simulink model "TosAeb"  (R2021a)
settings: Solver=FixedStepDiscrete  StartTime=0.0  StopTime=inf  FixedStep=0.01  RelTol=1e-3
undefined symbols (not defined in the file or in its folder): ACCModSts, AccSts1, AEB_ObjectMovingOrientation, AEB_ObjectMovingState, CN_BTCActive, CN_CurrentGearR, CN_CurrentGearR_DHT, CN_PTCActive, CN_VDCActive, CN_VSC_EspOff, CN_VSC_FCW_AEB_Error, CN_VSC_FCW_AEB_NoDispay, CN_VSC_FCW_AEB_PerfmDgdtn, CN_VSC_FCW_AEB_TempUnAvlbl, CN_VSC_VehStandstill, CNaccelAvoidMax, CNaccelAvoidMin, CNbrakePedalPress, CNcomfortStrLeftAccelMax, CNcomfortStrLeftAccelMin, CNcomfortStrRightAccelMax, CNcomfortStrRightAccelMin, CNDrvDoorOpen, CNDrvModeEco, CNDrvModeNorm, CNDrvModeOther, CNDrvModeSport, CNDrvSBRreminder, CNNull, CNobjInvalidID, CNPassDoorOpen, CNPassSBRreminder, CNPEBSAccelNegInf, CNPEBSAccelNull, CNPEBSTimeInf, CNPEBSTimeNull, CNSTOPMAX, CNSTOPNULL, CNsubjWidth, CNSwtOFF, CNSwtON, CNTTCMAX, CNTTCNULL, CNVehConfigAEBCycNone, CNVehConfigAEBNone, CNVehConfigAEBPedNone, CNVehConfigFCWNone, CNVehConfigJANone, CollisionSeverity, FALSE, FCWSnvty, JACycObjCalcInfoDefaultParams, JAPedObjCalcInfoDefaultParams, JAVehObjCalcInfoDefaultParams, latCycObjCalcInfoDefaultParams, latPedObjCalcInfoDefaultParams, longObjCalcInfoDefaultParams, ModSts, movingStatus, ObjectType ... (+1112)
libraries: simulink x3031, AEBSFunctionLib x50, Ta_Library x33, AEBSStateMachineLib x23, Mylibrary x12, simulink_extras x7, ObjSelect_lib x3, dspstat3 x2
(part 86 of 102)

== system TosAeb/AEB_Objsel/TA_Processor/AEB_ObjSelect/TargetInfoCheck/Data_precess/TA_EffectCalculate/Obj_FilterFlgCalculate/Long_ObjFilter/TTR_Calculate/CalculateTTRAndTTM_else/LongitudinalTTRComputer/CalculateTTI/StationaryHost/CheckIfSolutionExists ==
  note: This subsystem checks if there is a solution to the motion equation ARel*t^2/2 + VRel*t + EdgeDistance = 0.
      |
      | The cases with positive and negative ARel are handled separately.
      | If ARel == 0, there is always a solution if VRel ~= 0.
      | The case VRel == 0 and ARel == 0 is handled outside this subsystem.
  note: Same zero threshold as in the
      | quadratic equation solver.
  note: Solution exists if ARel == 0.
  EdgeDistance : Inport
  VRel : Inport  Port=2
  ARel : Inport  Port=3
  Compare To Zero : Subsystem  mask(Compare To Zero){relop=>, OutDataTypeStr=boolean, ZeroCross=on}
      mask prompts: relop "Simulink:masks:Operator_MP"; OutDataTypeStr "Simulink:masks:OutputDataType_MP"; ZeroCross "Simulink:masks:EnableZerocrossingDetection_MP"
      mask help: Simulink:masks:CompareToZero_MD
      mask init: same as TosAeb/AEB_Objsel/TA_Processor/AEB_ObjSelect/TargetInfoCheck/Data_precess/TA_EffectCalculate/Obj_FilterFlgCalculate/Long_ObjFilter/TTR_Calculate/CalculateTTRAndTTM_else/ModifyTTRAtSameSpeedAndAcceleration/Compare To Zero
      mask TypeOptions relop: == | ~= | < | <= | >= | >
      mask TypeOptions OutDataTypeStr: Simulink:masks:uint8 | Simulink:masks:boolean
      mask Callback ZeroCross: sl('zeroCrossEnable', gcbh);
      mask Display: disp([relop,' 0'])
  Compare To Zero1 : Subsystem  mask(Compare To Zero){relop=<, OutDataTypeStr=boolean, ZeroCross=on}
      mask prompts: relop "Simulink:masks:Operator_MP"; OutDataTypeStr "Simulink:masks:OutputDataType_MP"; ZeroCross "Simulink:masks:EnableZerocrossingDetection_MP"
      mask help: Simulink:masks:CompareToZero_MD
      mask init: same as TosAeb/AEB_Objsel/TA_Processor/AEB_ObjSelect/TargetInfoCheck/Data_precess/TA_EffectCalculate/Obj_FilterFlgCalculate/Long_ObjFilter/TTR_Calculate/CalculateTTRAndTTM_else/ModifyTTRAtSameSpeedAndAcceleration/Compare To Zero
      mask TypeOptions relop: == | ~= | < | <= | >= | >
      mask TypeOptions OutDataTypeStr: Simulink:masks:uint8 | Simulink:masks:boolean
      mask Callback ZeroCross: sl('zeroCrossEnable', gcbh);
      mask Display: disp([relop,' 0'])
  Constant : Constant  Value=false  OutDataTypeStr=boolean
  Gain : Gain  Gain=2
  LimitedSafeDivide : Subsystem
  Logop_1 : Logic  AllPortsSameDT=off  OutDataTypeStr=boolean
  Logop_2 : Logic  AllPortsSameDT=off  OutDataTypeStr=boolean
  Logop_3 : Logic  Operator=OR  AllPortsSameDT=off  OutDataTypeStr=boolean
  Relop_1 : RelationalOperator  Operator=>  OutDataTypeStr=boolean  RndMeth=Nearest
  Relop_2 : RelationalOperator  Operator=<  OutDataTypeStr=boolean  RndMeth=Nearest
  SizeChecker : Subsystem  mask(Size checker){UseInput=off, Limit=1e-2, DataTypeIn=single, Operator=>}
      mask prompts: UseInput "Take the limit as an input"; DataTypeIn "Data type:"; Operator "Relational operator:"
      mask help: The block tests the absolute value of the supplied signal against a specified value using the selected operator. If the signal conforms to the test, true is returned; else, false is returned. The limit can either be set in the mask or as an external signal.
      mask init: same as TosAeb/AEB_Objsel/TA_Processor/AEB_ObjSelect/TargetInfoCheck/Data_precess/TA_EffectCalculate/Obj_FilterFlgCalculate/Long_ObjFilter/TTR_Calculate/CalculateTTRAndTTM_else/ModifyTTRAtSameSpeedAndAcceleration/SizeChecker
      settings: AttributesFormatString=DT=%<DataTypeIn>
      mask Help: The block tests the absolute value of the supplied signal against a specified value using the selected operator. If the signal conforms to the test, true is returned; else, false is returned. The limit can either be set in the mask or as an external signal.
      mask Callback UseInput:
      | % Author: Jakob Andersson (jande573)
      | 
      | % Enable and disable the box for the limit variable depending on the status of the checkbox
      | if strcmp(get_param(gcb,'UseInput'),'on')
      |     % Disable the box
      |     set_param(gcb,'MaskEnables',{'on','off','on','on'}); % One setting for each parameter in this mask; needs to be updated if the parameters are changed
      | else
      |     % Enable the box
      |     set_param(gcb,'MaskEnables',{'on','on','on','on'}); % One setting for each parameter in this mask; needs to be updated if the parameters are changed
      | end
      mask Callback Limit:
      | % Author: Jakob Andersson (jande573)
      | 
      | % Define the default value to apply if the entered value is disallowed
      | default='1e-6';
      | 
      | % Evaluate the entered value
      | val=eval(get_param(gcb,'Limit'));
      | 
      | % Test the entered value and revert to the default if necessary
      | if isnumeric(val)==false
      |     % Set the default if the entered value did not evaluate to a numeric
      |     set_param(gcb,'Limit',default);
      |     errordlg('The limit must be numeric. The default value will be used instead.');
      | elseif val<0
      |     % Set the default if the entered value is negative
      |     set_param(gcb,'Limit',default);
      |     errordlg('The limit must be positive since it is compared to an absolute value. The default value will be used instead.');
      | end
      mask TypeOptions Operator:
      | Relational
      | Operator/Operator
      mask Callback Operator:
      | % Author: Jakob Andersson (jande573)
      | 
      | % Define the default value to apply if the entered value is disallowed
      | EnabledOperators={'==','~=','<','<=','>=','>'};
      | default='>';
      | 
      | % Evaluate the selected operator and determine if it is ok
      | ok=any(strcmp(get_param(gcb,'Operator'),EnabledOperators));
      | 
      | % Revert the operator if it is disabled
      | if ~ok
      |     % Set the default if the entered value did not evaluate to a numeric
      |     set_param(gcb,'Operator',default);
      |     errordlg('The selected operator is disabled. The default operator will be used instead.');
      | end
      mask Display:
      | % Display the mask for this block
      | % Author: Jakob Andersson (jande573)
      | 
      | % Transform the operator to TeX
      | switch Operator
      |     case '=='
      |         op='=';
      |     case '~='
      |         op='\neq';
      |     case '<='
      |         op='\leq';
      |     case '>='
      |         op='\geq';
      |     otherwise
      |         op=Operator;
      | end
      | 
      | % Display the correct mask depending on the number of inputs
      | if strcmp(UseInput,'on')
      |     % Display the block logic and the set limit
      |     disp(horzcat('|u| ',op,' a'),'texmode','on');
      | 
      |     % Display the port title
      |     port_label('input',1,'u');
      |     port_label('input',2,'a');
      | else
      |     % Display the block logic and the set limit
      |     disp(horzcat('|u| ',op,' ',num2str(Limit)),'texmode','on');
      | 
      |     % Display the port title
      |     port_label('input',1,'u');
      | end
  Square : Math  Operator=square  SignedPower=on  SaturateOnIntegerOverflow=on
  Switch : Switch  Criteria=u2 ~= 0
  NoSolution : Outport
  wires:
  Constant/1 -> Switch/3  "False"
  Switch/1 -> NoSolution/1  "NoSolution"
  SizeChecker/1(TestOk) -> Switch/2  "ARelIsDetectable"
  VRel/1 -> Square/1
  ARel/1 -> Compare To Zero/1(u), Compare To Zero1/1(u), SizeChecker/1(Signal), Gain/1
  EdgeDistance/1 -> Relop_1/1, Relop_2/1
  Square/1 -> LimitedSafeDivide/1(Numerator)
  Gain/1 -> LimitedSafeDivide/2(Denominator)
  LimitedSafeDivide/1(LimitedQuotient) -> Relop_1/2, Relop_2/2
  Compare To Zero/1(y) -> Logop_1/2  "PositiveARel"
  Relop_1/1 -> Logop_1/1
  Compare To Zero1/1(y) -> Logop_2/2  "NegativeARel"
  Logop_1/1 -> Logop_3/1  "NoSolutionPositiveARel"
  Logop_2/1 -> Logop_3/2  "NoSolutionNegativeARel"
  Logop_3/1 -> Switch/1  "NoSolutionCalculatedUsingARel"
  [BelowLimit] -> Logop_2/1
  Relop_2/1 -> [BelowLimit]

== system TosAeb/AEB_Objsel/TA_Processor/AEB_ObjSelect/TargetInfoCheck/Data_precess/TA_EffectCalculate/Obj_FilterFlgCalculate/Long_ObjFilter/TTR_Calculate/CalculateTTRAndTTM_else/LongitudinalTTRComputer/CalculateTTI/StationaryHost/CheckIfSolutionExists1 ==
  note: This subsystem checks if there is a solution to the motion equation ARel*t^2/2 + VRel*t + EdgeDistance = 0.
      |
      | The cases with positive and negative ARel are handled separately.
      | If ARel == 0, there is always a solution if VRel ~= 0.
      | The case VRel == 0 and ARel == 0 is handled outside this subsystem.
  note: Same zero threshold as in the
      | quadratic equation solver.
  note: Solution exists if ARel == 0.
  EdgeDistance : Inport
  VRel : Inport  Port=2
  ARel : Inport  Port=3
  Compare To Zero : Subsystem  mask(Compare To Zero){relop=>, OutDataTypeStr=boolean, ZeroCross=on}
      mask prompts: relop "Simulink:masks:Operator_MP"; OutDataTypeStr "Simulink:masks:OutputDataType_MP"; ZeroCross "Simulink:masks:EnableZerocrossingDetection_MP"
      mask help: Simulink:masks:CompareToZero_MD
      mask init: same as TosAeb/AEB_Objsel/TA_Processor/AEB_ObjSelect/TargetInfoCheck/Data_precess/TA_EffectCalculate/Obj_FilterFlgCalculate/Long_ObjFilter/TTR_Calculate/CalculateTTRAndTTM_else/ModifyTTRAtSameSpeedAndAcceleration/Compare To Zero
      mask TypeOptions relop: == | ~= | < | <= | >= | >
      mask TypeOptions OutDataTypeStr: Simulink:masks:uint8 | Simulink:masks:boolean
      mask Callback ZeroCross: sl('zeroCrossEnable', gcbh);
      mask Display: disp([relop,' 0'])
  Compare To Zero1 : Subsystem  mask(Compare To Zero){relop=<, OutDataTypeStr=boolean, ZeroCross=on}
      mask prompts: relop "Simulink:masks:Operator_MP"; OutDataTypeStr "Simulink:masks:OutputDataType_MP"; ZeroCross "Simulink:masks:EnableZerocrossingDetection_MP"
      mask help: Simulink:masks:CompareToZero_MD
      mask init: same as TosAeb/AEB_Objsel/TA_Processor/AEB_ObjSelect/TargetInfoCheck/Data_precess/TA_EffectCalculate/Obj_FilterFlgCalculate/Long_ObjFilter/TTR_Calculate/CalculateTTRAndTTM_else/ModifyTTRAtSameSpeedAndAcceleration/Compare To Zero
      mask TypeOptions relop: == | ~= | < | <= | >= | >
      mask TypeOptions OutDataTypeStr: Simulink:masks:uint8 | Simulink:masks:boolean
      mask Callback ZeroCross: sl('zeroCrossEnable', gcbh);
      mask Display: disp([relop,' 0'])
  Constant : Constant  Value=false  OutDataTypeStr=boolean
  Gain : Gain  Gain=2
  LimitedSafeDivide : Subsystem
  Logop_1 : Logic  AllPortsSameDT=off  OutDataTypeStr=boolean
  Logop_2 : Logic  AllPortsSameDT=off  OutDataTypeStr=boolean
  Logop_3 : Logic  Operator=OR  AllPortsSameDT=off  OutDataTypeStr=boolean
  Relop_1 : RelationalOperator  Operator=>  OutDataTypeStr=boolean  RndMeth=Nearest
  Relop_2 : RelationalOperator  Operator=<  OutDataTypeStr=boolean  RndMeth=Nearest
  SizeChecker : Subsystem  mask(Size checker){UseInput=off, Limit=1e-2, DataTypeIn=single, Operator=>}
      mask prompts: UseInput "Take the limit as an input"; DataTypeIn "Data type:"; Operator "Relational operator:"
      mask help: The block tests the absolute value of the supplied signal against a specified value using the selected operator. If the signal conforms to the test, true is returned; else, false is returned. The limit can either be set in the mask or as an external signal.
      mask init: same as TosAeb/AEB_Objsel/TA_Processor/AEB_ObjSelect/TargetInfoCheck/Data_precess/TA_EffectCalculate/Obj_FilterFlgCalculate/Long_ObjFilter/TTR_Calculate/CalculateTTRAndTTM_else/ModifyTTRAtSameSpeedAndAcceleration/SizeChecker
      settings: AttributesFormatString=DT=%<DataTypeIn>
      mask Help: The block tests the absolute value of the supplied signal against a specified value using the selected operator. If the signal conforms to the test, true is returned; else, false is returned. The limit can either be set in the mask or as an external signal.
      mask Callback UseInput:
      | % Author: Jakob Andersson (jande573)
      | 
      | % Enable and disable the box for the limit variable depending on the status of the checkbox
      | if strcmp(get_param(gcb,'UseInput'),'on')
      |     % Disable the box
      |     set_param(gcb,'MaskEnables',{'on','off','on','on'}); % One setting for each parameter in this mask; needs to be updated if the parameters are changed
      | else
      |     % Enable the box
      |     set_param(gcb,'MaskEnables',{'on','on','on','on'}); % One setting for each parameter in this mask; needs to be updated if the parameters are changed
      | end
      mask Callback Limit:
      | % Author: Jakob Andersson (jande573)
      | 
      | % Define the default value to apply if the entered value is disallowed
      | default='1e-6';
      | 
      | % Evaluate the entered value
      | val=eval(get_param(gcb,'Limit'));
      | 
      | % Test the entered value and revert to the default if necessary
      | if isnumeric(val)==false
      |     % Set the default if the entered value did not evaluate to a numeric
      |     set_param(gcb,'Limit',default);
      |     errordlg('The limit must be numeric. The default value will be used instead.');
      | elseif val<0
      |     % Set the default if the entered value is negative
      |     set_param(gcb,'Limit',default);
      |     errordlg('The limit must be positive since it is compared to an absolute value. The default value will be used instead.');
      | end
      mask TypeOptions Operator:
      | Relational
      | Operator/Operator
      mask Callback Operator:
      | % Author: Jakob Andersson (jande573)
      | 
      | % Define the default value to apply if the entered value is disallowed
      | EnabledOperators={'==','~=','<','<=','>=','>'};
      | default='>';
      | 
      | % Evaluate the selected operator and determine if it is ok
      | ok=any(strcmp(get_param(gcb,'Operator'),EnabledOperators));
      | 
      | % Revert the operator if it is disabled
      | if ~ok
      |     % Set the default if the entered value did not evaluate to a numeric
      |     set_param(gcb,'Operator',default);
      |     errordlg('The selected operator is disabled. The default operator will be used instead.');
      | end
      mask Display:
      | % Display the mask for this block
      | % Author: Jakob Andersson (jande573)
      | 
      | % Transform the operator to TeX
      | switch Operator
      |     case '=='
      |         op='=';
      |     case '~='
      |         op='\neq';
      |     case '<='
      |         op='\leq';
      |     case '>='
      |         op='\geq';
      |     otherwise
      |         op=Operator;
      | end
      | 
      | % Display the correct mask depending on the number of inputs
      | if strcmp(UseInput,'on')
      |     % Display the block logic and the set limit
      |     disp(horzcat('|u| ',op,' a'),'texmode','on');
      | 
      |     % Display the port title
      |     port_label('input',1,'u');
      |     port_label('input',2,'a');
      | else
      |     % Display the block logic and the set limit
      |     disp(horzcat('|u| ',op,' ',num2str(Limit)),'texmode','on');
      | 
      |     % Display the port title
      |     port_label('input',1,'u');
      | end
  Square : Math  Operator=square  SignedPower=on  SaturateOnIntegerOverflow=on
  Switch : Switch  Criteria=u2 ~= 0
  NoSolution : Outport
  wires:
  Constant/1 -> Switch/3  "False"
  Switch/1 -> NoSolution/1  "NoSolution"
  SizeChecker/1(TestOk) -> Switch/2  "ARelIsDetectable"
  VRel/1 -> Square/1
  ARel/1 -> Compare To Zero/1(u), Compare To Zero1/1(u), SizeChecker/1(Signal), Gain/1
  EdgeDistance/1 -> Relop_1/1, Relop_2/1
  Square/1 -> LimitedSafeDivide/1(Numerator)
  Gain/1 -> LimitedSafeDivide/2(Denominator)
  LimitedSafeDivide/1(LimitedQuotient) -> Relop_1/2, Relop_2/2
  Compare To Zero/1(y) -> Logop_1/2  "PositiveARel"
  Relop_1/1 -> Logop_1/1
  Compare To Zero1/1(y) -> Logop_2/2  "NegativeARel"
  Logop_1/1 -> Logop_3/1  "NoSolutionPositiveARel"
  Logop_2/1 -> Logop_3/2  "NoSolutionNegativeARel"
  Logop_3/1 -> Switch/1  "NoSolutionCalculatedUsingARel"
  [BelowLimit] -> Logop_2/1
  Relop_2/1 -> [BelowLimit]

== system TosAeb/AEB_Objsel/TA_Processor/AEB_ObjSelect/TargetInfoCheck/Data_precess/TA_EffectCalculate/Obj_FilterFlgCalculate/Long_ObjFilter/TTR_Calculate/CalculateTTRAndTTM_else/LongitudinalTTRComputer/CalculateTTI/StationaryHost/TimeToIntersectionComputer ==
  note: 求解一元二次方程，并判断是否存在解、
      | 依据位置关系进行判断输出TTI数值
  note: 100
  note: -100
  note: a*t^2/2 + v*t + x = 0
  VRel : Inport  OutDataTypeStr=single
      settings: AttributesFormatString=DT=%<OutDataTypeStr>
  ARel : Inport  Port=2  OutDataTypeStr=single
      settings: AttributesFormatString=DT=%<OutDataTypeStr>
  Posn : Inport  Port=3  OutDataTypeStr=single
      settings: AttributesFormatString=DT=%<OutDataTypeStr>
  AEB_State : Inport  Port=4
  Abs : Abs
  Abs1 : Abs
  Compare To Constant : simulink/Logic and Bit Operations/Compare To Constant  relop=<=  const=0.25  OutDataTypeStr=boolean
  Compare To Constant1 : simulink/Logic and Bit Operations/Compare To Constant  relop===  const=0  OutDataTypeStr=boolean
  Compare To Constant10 : simulink/Logic and Bit Operations/Compare To Constant  relop===  const=0  OutDataTypeStr=boolean
  Compare To Constant2 : simulink/Logic and Bit Operations/Compare To Constant  relop=~=  const=0  OutDataTypeStr=boolean
  Compare To Constant3 : simulink/Logic and Bit Operations/Compare To Constant  relop===  const=0  OutDataTypeStr=boolean
  Compare To Constant4 : simulink/Logic and Bit Operations/Compare To Constant  relop=~=  const=100  OutDataTypeStr=boolean
  Compare To Constant5 : simulink/Logic and Bit Operations/Compare To Constant  relop=~=  const=-100  OutDataTypeStr=boolean
  Compare To Constant7 : simulink/Logic and Bit Operations/Compare To Constant  relop=~=  const=0  OutDataTypeStr=boolean
  Compare To Constant8 : simulink/Logic and Bit Operations/Compare To Constant  relop=<=  const=6  OutDataTypeStr=boolean
  Compare To Constant9 : simulink/Logic and Bit Operations/Compare To Constant  relop=<=  const=6  OutDataTypeStr=boolean
  Compare To Zero : Subsystem  mask(Compare To Zero){relop=<, OutDataTypeStr=boolean, ZeroCross=on}
      mask prompts: relop "Simulink:masks:Operator_MP"; OutDataTypeStr "Simulink:masks:OutputDataType_MP"; ZeroCross "Simulink:masks:EnableZerocrossingDetection_MP"
      mask help: Simulink:masks:CompareToZero_MD
      mask init: same as TosAeb/AEB_Objsel/TA_Processor/AEB_ObjSelect/TargetInfoCheck/Data_precess/TA_EffectCalculate/Obj_FilterFlgCalculate/Long_ObjFilter/TTR_Calculate/CalculateTTRAndTTM_else/ModifyTTRAtSameSpeedAndAcceleration/Compare To Zero
      mask TypeOptions relop: == | ~= | < | <= | >= | >
      mask TypeOptions OutDataTypeStr: Simulink:masks:uint8 | Simulink:masks:boolean
      mask Callback ZeroCross: sl('zeroCrossEnable', gcbh);
      mask Display: disp([relop,' 0'])
  Constant : Constant  OutDataTypeStr=single
  Constant1 : Constant  Value=P_TA_TiLowrLimInAsyEvlrCritEve  OutDataTypeStr=single
      settings: AttributesFormatString=DT=%<OutDataTypeStr>
  Constant3 : Constant  Value=P_TA_TiUpprLimInAsyEvlrCritEve  OutDataTypeStr=single
      settings: AttributesFormatString=DT=%<OutDataTypeStr>
  Delay : Delay  DelayLength=5
  Divide : Product  Inputs=*/
  Gain : Gain  Gain=0.5
  Logical Operator : Logic  Operator=OR  Inputs=3  AllPortsSameDT=off  OutDataTypeStr=boolean
  Logical Operator1 : Logic  Inputs=3  AllPortsSameDT=off  OutDataTypeStr=boolean
  Logical Operator2 : Logic  AllPortsSameDT=off  OutDataTypeStr=boolean
  Logical Operator3 : Logic  Inputs=3  AllPortsSameDT=off  OutDataTypeStr=boolean
  QuadraticEquationSolver : MATLAB Function  TreatAsAtomicUnit=on  RTWFileName=QuadraticEquationSolverNew  mask(Quadratic equation solver){ZeroThreshold=single(1e-2)}
      mask prompts: ZeroThreshold "Zero threshold:"
      mask help:
      | This block solves a quadratic polynomial equation.
      | Both solutions are returned in a bus.
      | If the equation lacks real roots then zeros are returned,
      | along with a flag indicating that no solutions where found.
      settings: RTWFcnNameOpts=Use subsystem name
  Relational Operator : RelationalOperator  Operator=<=  OutDataTypeStr=boolean  RndMeth=Simplest
  SolutionChooser : Subsystem
  Switch : Switch  Criteria=u2 > Threshold
  Switch1 : Switch  Criteria=u2 ~= 0  InputSameDT=on
      settings: AttributesFormatString=InputSameDT=%<InputSameDT>
  Switch2 : Switch  Criteria=u2 ~= 0  InputSameDT=on
      settings: AttributesFormatString=InputSameDT=%<InputSameDT>
  Switch3 : Switch  Criteria=u2 > Threshold
  Switch4 : Switch  Criteria=u2 > Threshold
  Terminator1 : Terminator
  Unit Delay : UnitDelay  SampleTime=-1  HasFrameUpgradeWarning=on
  TimeToIntersection : Outport  OutDataTypeStr=single
      settings: AttributesFormatString=DT=%<OutDataTypeStr>
  wires:
  Switch1/1 -> Switch2/3  "DefaultTTI"
  Constant1/1 -> Switch1/1  "DefaultNegativeTTI"
  Compare To Zero/1(y) -> Switch1/2
  SolutionChooser/1(Solution) -> Switch2/1
  QuadraticEquationSolver/2(SolutionFound) -> [Solution], Switch2/2
  QuadraticEquationSolver/1(x) -> SolutionChooser/1(Solutions)
  Posn/1 -> [B], Divide/1, QuadraticEquationSolver/3(c), Compare To Zero/1(u)  "Posn"
  VRel/1 -> QuadraticEquationSolver/2(b), [VRel]  "VRel"
  Switch2/1 -> Switch/3  "TimeToIntersection"
  Constant3/1 -> Switch1/3  "DefaultPositiveTTI"
  Gain/1 -> QuadraticEquationSolver/1(a)  "ARelHalf"
  [ARel] -> SolutionChooser/2(ARel)
  [ARel] -> Abs1/1
  [Solution] -> Compare To Constant1/1
  Compare To Constant/1 -> Logical Operator/1
  Compare To Constant1/1 -> Logical Operator/2
  Logical Operator/1 -> Switch/2
  Compare To Constant3/1 -> Switch3/2
  Constant/1 -> Switch3/1
  [VRel] -> Abs/1, Compare To Constant3/1
  Switch3/1 -> Divide/2
  Abs/1 -> Switch3/3
  Divide/1 -> [A]
  [A] -> Switch/1
  Switch4/1 -> TimeToIntersection/1, Unit Delay/1
  Abs1/1 -> Compare To Constant/1
  Unit Delay/1 -> Compare To Constant8/1, Compare To Constant5/1, Compare To Constant4/1, Switch4/1
  [A] -> Compare To Constant9/1, Compare To Constant7/1
  [B] -> Delay/1, Relational Operator/1
  Compare To Constant4/1 -> Logical Operator1/1
  Compare To Constant5/1 -> Logical Operator1/2
  Compare To Constant8/1 -> Logical Operator1/3
  [Solution] -> Compare To Constant10/1
  Delay/1 -> Relational Operator/2
  Compare To Constant7/1 -> Logical Operator2/1
  Compare To Constant9/1 -> Logical Operator2/2
  Logical Operator1/1 -> Logical Operator3/1
  Logical Operator2/1 -> Logical Operator3/2
  Relational Operator/1 -> Terminator1/1
  Logical Operator3/1 -> Switch4/2
  Switch/1 -> Switch4/3
  Compare To Constant10/1 -> Logical Operator3/3
  AEB_State/1 -> Compare To Constant2/1
  Compare To Constant2/1 -> Logical Operator/3
  ARel/1 -> [ARel], Gain/1  "ARel"

== system TosAeb/AEB_Objsel/TA_Processor/AEB_ObjSelect/TargetInfoCheck/Data_precess/TA_EffectCalculate/Obj_FilterFlgCalculate/Long_ObjFilter/TTR_Calculate/CalculateTTRAndTTM_else/LongitudinalTTRComputer/CalculateTTI/StationaryHost/TimeToIntersectionComputer1 ==
  note: a*t^2/2 + v*t + x = 0
  note: -100
  note: 100
  note: 求解一元二次方程，并判断是否存在解、
      | 依据位置关系进行判断输出TTI数值
  VRel : Inport  OutDataTypeStr=single
      settings: AttributesFormatString=DT=%<OutDataTypeStr>
  ARel : Inport  Port=2  OutDataTypeStr=single
      settings: AttributesFormatString=DT=%<OutDataTypeStr>
  Posn : Inport  Port=3  OutDataTypeStr=single
      settings: AttributesFormatString=DT=%<OutDataTypeStr>
  Abs : Abs
  Abs1 : Abs
  Compare To Constant : simulink/Logic and Bit Operations/Compare To Constant  relop=<=  const=0.25  OutDataTypeStr=boolean
  Compare To Constant1 : simulink/Logic and Bit Operations/Compare To Constant  relop===  const=0  OutDataTypeStr=boolean
  Compare To Constant2 : simulink/Logic and Bit Operations/Compare To Constant  relop=<  const=0  OutDataTypeStr=boolean
  Compare To Constant3 : simulink/Logic and Bit Operations/Compare To Constant  relop===  const=0  OutDataTypeStr=boolean
  Compare To Zero : Subsystem  mask(Compare To Zero){relop=<, OutDataTypeStr=boolean, ZeroCross=on}
      mask prompts: relop "Simulink:masks:Operator_MP"; OutDataTypeStr "Simulink:masks:OutputDataType_MP"; ZeroCross "Simulink:masks:EnableZerocrossingDetection_MP"
      mask help: Simulink:masks:CompareToZero_MD
      mask init: same as TosAeb/AEB_Objsel/TA_Processor/AEB_ObjSelect/TargetInfoCheck/Data_precess/TA_EffectCalculate/Obj_FilterFlgCalculate/Long_ObjFilter/TTR_Calculate/CalculateTTRAndTTM_else/ModifyTTRAtSameSpeedAndAcceleration/Compare To Zero
      mask TypeOptions relop: == | ~= | < | <= | >= | >
      mask TypeOptions OutDataTypeStr: Simulink:masks:uint8 | Simulink:masks:boolean
      mask Callback ZeroCross: sl('zeroCrossEnable', gcbh);
      mask Display: disp([relop,' 0'])
  Constant : Constant  OutDataTypeStr=single
  Constant1 : Constant  Value=P_TA_TiLowrLimInAsyEvlrCritEve  OutDataTypeStr=single
      settings: AttributesFormatString=DT=%<OutDataTypeStr>
  Constant3 : Constant  Value=P_TA_TiUpprLimInAsyEvlrCritEve  OutDataTypeStr=single
      settings: AttributesFormatString=DT=%<OutDataTypeStr>
  Divide : Product  Inputs=*/
  Gain : Gain  Gain=0.5
  Logical Operator : Logic  Inputs=3  AllPortsSameDT=off  OutDataTypeStr=boolean
  QuadraticEquationSolver : MATLAB Function  TreatAsAtomicUnit=on  RTWFileName=QuadraticEquationSolverNew  mask(Quadratic equation solver){ZeroThreshold=single(1e-2)}
      mask prompts: ZeroThreshold "Zero threshold:"
      mask help:
      | This block solves a quadratic polynomial equation.
      | Both solutions are returned in a bus.
      | If the equation lacks real roots then zeros are returned,
      | along with a flag indicating that no solutions where found.
      settings: RTWFcnNameOpts=Use subsystem name
  SolutionChooser : Subsystem
  Switch : Switch  Criteria=u2 > Threshold
  Switch1 : Switch  Criteria=u2 ~= 0  InputSameDT=on
      settings: AttributesFormatString=InputSameDT=%<InputSameDT>
  Switch2 : Switch  Criteria=u2 ~= 0  InputSameDT=on
      settings: AttributesFormatString=InputSameDT=%<InputSameDT>
  Switch3 : Switch  Criteria=u2 > Threshold
  TimeToIntersection : Outport  OutDataTypeStr=single
      settings: AttributesFormatString=DT=%<OutDataTypeStr>
  wires:
  Abs1/1 -> Compare To Constant/1
  Switch/1 -> TimeToIntersection/1
  [A] -> Switch/1
  Divide/1 -> [A]
  Abs/1 -> Switch3/3
  Switch3/1 -> Divide/2
  [VRel] -> Compare To Constant3/1, Abs/1
  Constant/1 -> Switch3/1
  Compare To Constant3/1 -> Switch3/2
  Compare To Constant2/1 -> Logical Operator/3
  [VRel] -> Compare To Constant2/1
  Logical Operator/1 -> Switch/2
  Compare To Constant1/1 -> Logical Operator/2
  Compare To Constant/1 -> Logical Operator/1
  [Solution] -> Compare To Constant1/1
  [ARel] -> Abs1/1
  [ARel] -> SolutionChooser/2(ARel)
  Gain/1 -> QuadraticEquationSolver/1(a)  "ARelHalf"
  Constant3/1 -> Switch1/3  "DefaultPositiveTTI"
  Switch2/1 -> Switch/3  "TimeToIntersection"
  VRel/1 -> [VRel], QuadraticEquationSolver/2(b)  "VRel"
  Posn/1 -> Compare To Zero/1(u), QuadraticEquationSolver/3(c), Divide/1  "Posn"
  ARel/1 -> [ARel], Gain/1  "ARel"
  QuadraticEquationSolver/1(x) -> SolutionChooser/1(Solutions)
  QuadraticEquationSolver/2(SolutionFound) -> Switch2/2, [Solution]
  SolutionChooser/1(Solution) -> Switch2/1
  Compare To Zero/1(y) -> Switch1/2
  Constant1/1 -> Switch1/1  "DefaultNegativeTTI"
  Switch1/1 -> Switch2/3  "DefaultTTI"

== system TosAeb/AEB_Objsel/TA_Processor/AEB_ObjSelect/TargetInfoCheck/Data_precess/TA_EffectCalculate/Obj_FilterFlgCalculate/Long_ObjFilter/TTR_Calculate/CalculateTTRAndTTM_else/LongitudinalTTRComputer/CalculateTTI/StationaryObject/CheckIfSolutionExists ==
  note: This subsystem checks if there is a solution to the motion equation ARel*t^2/2 + VRel*t + EdgeDistance = 0.
      |
      | The cases with positive and negative ARel are handled separately.
      | If ARel == 0, there is always a solution if VRel ~= 0.
      | The case VRel == 0 and ARel == 0 is handled outside this subsystem.
  note: Same zero threshold as in the
      | quadratic equation solver.
  note: Solution exists if ARel == 0.
  EdgeDistance : Inport
  VRel : Inport  Port=2
  ARel : Inport  Port=3
  Compare To Zero : Subsystem  mask(Compare To Zero){relop=>, OutDataTypeStr=boolean, ZeroCross=on}
      mask prompts: relop "Simulink:masks:Operator_MP"; OutDataTypeStr "Simulink:masks:OutputDataType_MP"; ZeroCross "Simulink:masks:EnableZerocrossingDetection_MP"
      mask help: Simulink:masks:CompareToZero_MD
      mask init: same as TosAeb/AEB_Objsel/TA_Processor/AEB_ObjSelect/TargetInfoCheck/Data_precess/TA_EffectCalculate/Obj_FilterFlgCalculate/Long_ObjFilter/TTR_Calculate/CalculateTTRAndTTM_else/ModifyTTRAtSameSpeedAndAcceleration/Compare To Zero
      mask TypeOptions relop: == | ~= | < | <= | >= | >
      mask TypeOptions OutDataTypeStr: Simulink:masks:uint8 | Simulink:masks:boolean
      mask Callback ZeroCross: sl('zeroCrossEnable', gcbh);
      mask Display: disp([relop,' 0'])
  Compare To Zero1 : Subsystem  mask(Compare To Zero){relop=<, OutDataTypeStr=boolean, ZeroCross=on}
      mask prompts: relop "Simulink:masks:Operator_MP"; OutDataTypeStr "Simulink:masks:OutputDataType_MP"; ZeroCross "Simulink:masks:EnableZerocrossingDetection_MP"
      mask help: Simulink:masks:CompareToZero_MD
      mask init: same as TosAeb/AEB_Objsel/TA_Processor/AEB_ObjSelect/TargetInfoCheck/Data_precess/TA_EffectCalculate/Obj_FilterFlgCalculate/Long_ObjFilter/TTR_Calculate/CalculateTTRAndTTM_else/ModifyTTRAtSameSpeedAndAcceleration/Compare To Zero
      mask TypeOptions relop: == | ~= | < | <= | >= | >
      mask TypeOptions OutDataTypeStr: Simulink:masks:uint8 | Simulink:masks:boolean
      mask Callback ZeroCross: sl('zeroCrossEnable', gcbh);
      mask Display: disp([relop,' 0'])
  Constant : Constant  Value=false  OutDataTypeStr=boolean
  Gain : Gain  Gain=2
  LimitedSafeDivide : Subsystem
  Logop_1 : Logic  AllPortsSameDT=off  OutDataTypeStr=boolean
  Logop_2 : Logic  AllPortsSameDT=off  OutDataTypeStr=boolean
  Logop_3 : Logic  Operator=OR  AllPortsSameDT=off  OutDataTypeStr=boolean
  Relop_1 : RelationalOperator  Operator=>  OutDataTypeStr=boolean  RndMeth=Nearest
  Relop_2 : RelationalOperator  Operator=<  OutDataTypeStr=boolean  RndMeth=Nearest
  SizeChecker : Subsystem  mask(Size checker){UseInput=off, Limit=1e-2, DataTypeIn=single, Operator=>}
      mask prompts: UseInput "Take the limit as an input"; DataTypeIn "Data type:"; Operator "Relational operator:"
      mask help: The block tests the absolute value of the supplied signal against a specified value using the selected operator. If the signal conforms to the test, true is returned; else, false is returned. The limit can either be set in the mask or as an external signal.
      mask init: same as TosAeb/AEB_Objsel/TA_Processor/AEB_ObjSelect/TargetInfoCheck/Data_precess/TA_EffectCalculate/Obj_FilterFlgCalculate/Long_ObjFilter/TTR_Calculate/CalculateTTRAndTTM_else/ModifyTTRAtSameSpeedAndAcceleration/SizeChecker
      settings: AttributesFormatString=DT=%<DataTypeIn>
      mask Help: The block tests the absolute value of the supplied signal against a specified value using the selected operator. If the signal conforms to the test, true is returned; else, false is returned. The limit can either be set in the mask or as an external signal.
      mask Callback UseInput:
      | % Author: Jakob Andersson (jande573)
      | 
      | % Enable and disable the box for the limit variable depending on the status of the checkbox
      | if strcmp(get_param(gcb,'UseInput'),'on')
      |     % Disable the box
      |     set_param(gcb,'MaskEnables',{'on','off','on','on'}); % One setting for each parameter in this mask; needs to be updated if the parameters are changed
      | else
      |     % Enable the box
      |     set_param(gcb,'MaskEnables',{'on','on','on','on'}); % One setting for each parameter in this mask; needs to be updated if the parameters are changed
      | end
      mask Callback Limit:
      | % Author: Jakob Andersson (jande573)
      | 
      | % Define the default value to apply if the entered value is disallowed
      | default='1e-6';
      | 
      | % Evaluate the entered value
      | val=eval(get_param(gcb,'Limit'));
      | 
      | % Test the entered value and revert to the default if necessary
      | if isnumeric(val)==false
      |     % Set the default if the entered value did not evaluate to a numeric
      |     set_param(gcb,'Limit',default);
      |     errordlg('The limit must be numeric. The default value will be used instead.');
      | elseif val<0
      |     % Set the default if the entered value is negative
      |     set_param(gcb,'Limit',default);
      |     errordlg('The limit must be positive since it is compared to an absolute value. The default value will be used instead.');
      | end
      mask TypeOptions Operator:
      | Relational
      | Operator/Operator
      mask Callback Operator:
      | % Author: Jakob Andersson (jande573)
      | 
      | % Define the default value to apply if the entered value is disallowed
      | EnabledOperators={'==','~=','<','<=','>=','>'};
      | default='>';
      | 
      | % Evaluate the selected operator and determine if it is ok
      | ok=any(strcmp(get_param(gcb,'Operator'),EnabledOperators));
      | 
      | % Revert the operator if it is disabled
      | if ~ok
      |     % Set the default if the entered value did not evaluate to a numeric
      |     set_param(gcb,'Operator',default);
      |     errordlg('The selected operator is disabled. The default operator will be used instead.');
      | end
      mask Display:
      | % Display the mask for this block
      | % Author: Jakob Andersson (jande573)
      | 
      | % Transform the operator to TeX
      | switch Operator
      |     case '=='
      |         op='=';
      |     case '~='
      |         op='\neq';
      |     case '<='
      |         op='\leq';
      |     case '>='
      |         op='\geq';
      |     otherwise
      |         op=Operator;
      | end
      | 
      | % Display the correct mask depending on the number of inputs
      | if strcmp(UseInput,'on')
      |     % Display the block logic and the set limit
      |     disp(horzcat('|u| ',op,' a'),'texmode','on');
      | 
      |     % Display the port title
      |     port_label('input',1,'u');
      |     port_label('input',2,'a');
      | else
      |     % Display the block logic and the set limit
      |     disp(horzcat('|u| ',op,' ',num2str(Limit)),'texmode','on');
      | 
      |     % Display the port title
      |     port_label('input',1,'u');
      | end
  Square : Math  Operator=square  SignedPower=on  SaturateOnIntegerOverflow=on
  Switch : Switch  Criteria=u2 ~= 0
  NoSolution : Outport
  wires:
  Constant/1 -> Switch/3  "False"
  Switch/1 -> NoSolution/1  "NoSolution"
  SizeChecker/1(TestOk) -> Switch/2  "ARelIsDetectable"
  VRel/1 -> Square/1
  ARel/1 -> Compare To Zero/1(u), Compare To Zero1/1(u), SizeChecker/1(Signal), Gain/1
  EdgeDistance/1 -> Relop_1/1, Relop_2/1
  Square/1 -> LimitedSafeDivide/1(Numerator)
  Gain/1 -> LimitedSafeDivide/2(Denominator)
  LimitedSafeDivide/1(LimitedQuotient) -> Relop_1/2, Relop_2/2
  Compare To Zero/1(y) -> Logop_1/2  "PositiveARel"
  Relop_1/1 -> Logop_1/1
  Compare To Zero1/1(y) -> Logop_2/2  "NegativeARel"
  Logop_1/1 -> Logop_3/1  "NoSolutionPositiveARel"
  Logop_2/1 -> Logop_3/2  "NoSolutionNegativeARel"
  Logop_3/1 -> Switch/1  "NoSolutionCalculatedUsingARel"
  [BelowLimit] -> Logop_2/1
  Relop_2/1 -> [BelowLimit]

== system TosAeb/AEB_Objsel/TA_Processor/AEB_ObjSelect/TargetInfoCheck/Data_precess/TA_EffectCalculate/Obj_FilterFlgCalculate/Long_ObjFilter/TTR_Calculate/CalculateTTRAndTTM_else/LongitudinalTTRComputer/CalculateTTI/StationaryObject/CheckIfSolutionExists1 ==
  note: This subsystem checks if there is a solution to the motion equation ARel*t^2/2 + VRel*t + EdgeDistance = 0.
      |
      | The cases with positive and negative ARel are handled separately.
      | If ARel == 0, there is always a solution if VRel ~= 0.
      | The case VRel == 0 and ARel == 0 is handled outside this subsystem.
  note: Same zero threshold as in the
      | quadratic equation solver.
  note: Solution exists if ARel == 0.
  EdgeDistance : Inport
  VRel : Inport  Port=2
  ARel : Inport  Port=3
  Compare To Zero : Subsystem  mask(Compare To Zero){relop=>, OutDataTypeStr=boolean, ZeroCross=on}
      mask prompts: relop "Simulink:masks:Operator_MP"; OutDataTypeStr "Simulink:masks:OutputDataType_MP"; ZeroCross "Simulink:masks:EnableZerocrossingDetection_MP"
      mask help: Simulink:masks:CompareToZero_MD
      mask init: same as TosAeb/AEB_Objsel/TA_Processor/AEB_ObjSelect/TargetInfoCheck/Data_precess/TA_EffectCalculate/Obj_FilterFlgCalculate/Long_ObjFilter/TTR_Calculate/CalculateTTRAndTTM_else/ModifyTTRAtSameSpeedAndAcceleration/Compare To Zero
      mask TypeOptions relop: == | ~= | < | <= | >= | >
      mask TypeOptions OutDataTypeStr: Simulink:masks:uint8 | Simulink:masks:boolean
      mask Callback ZeroCross: sl('zeroCrossEnable', gcbh);
      mask Display: disp([relop,' 0'])
  Compare To Zero1 : Subsystem  mask(Compare To Zero){relop=<, OutDataTypeStr=boolean, ZeroCross=on}
      mask prompts: relop "Simulink:masks:Operator_MP"; OutDataTypeStr "Simulink:masks:OutputDataType_MP"; ZeroCross "Simulink:masks:EnableZerocrossingDetection_MP"
      mask help: Simulink:masks:CompareToZero_MD
      mask init: same as TosAeb/AEB_Objsel/TA_Processor/AEB_ObjSelect/TargetInfoCheck/Data_precess/TA_EffectCalculate/Obj_FilterFlgCalculate/Long_ObjFilter/TTR_Calculate/CalculateTTRAndTTM_else/ModifyTTRAtSameSpeedAndAcceleration/Compare To Zero
      mask TypeOptions relop: == | ~= | < | <= | >= | >
      mask TypeOptions OutDataTypeStr: Simulink:masks:uint8 | Simulink:masks:boolean
      mask Callback ZeroCross: sl('zeroCrossEnable', gcbh);
      mask Display: disp([relop,' 0'])
  Constant : Constant  Value=false  OutDataTypeStr=boolean
  Gain : Gain  Gain=2
  LimitedSafeDivide : Subsystem
  Logop_1 : Logic  AllPortsSameDT=off  OutDataTypeStr=boolean
  Logop_2 : Logic  AllPortsSameDT=off  OutDataTypeStr=boolean
  Logop_3 : Logic  Operator=OR  AllPortsSameDT=off  OutDataTypeStr=boolean
  Relop_1 : RelationalOperator  Operator=>  OutDataTypeStr=boolean  RndMeth=Nearest
  Relop_2 : RelationalOperator  Operator=<  OutDataTypeStr=boolean  RndMeth=Nearest
  SizeChecker : Subsystem  mask(Size checker){UseInput=off, Limit=1e-2, DataTypeIn=single, Operator=>}
      mask prompts: UseInput "Take the limit as an input"; DataTypeIn "Data type:"; Operator "Relational operator:"
      mask help: The block tests the absolute value of the supplied signal against a specified value using the selected operator. If the signal conforms to the test, true is returned; else, false is returned. The limit can either be set in the mask or as an external signal.
      mask init: same as TosAeb/AEB_Objsel/TA_Processor/AEB_ObjSelect/TargetInfoCheck/Data_precess/TA_EffectCalculate/Obj_FilterFlgCalculate/Long_ObjFilter/TTR_Calculate/CalculateTTRAndTTM_else/ModifyTTRAtSameSpeedAndAcceleration/SizeChecker
      settings: AttributesFormatString=DT=%<DataTypeIn>
      mask Help: The block tests the absolute value of the supplied signal against a specified value using the selected operator. If the signal conforms to the test, true is returned; else, false is returned. The limit can either be set in the mask or as an external signal.
      mask Callback UseInput:
      | % Author: Jakob Andersson (jande573)
      | 
      | % Enable and disable the box for the limit variable depending on the status of the checkbox
      | if strcmp(get_param(gcb,'UseInput'),'on')
      |     % Disable the box
      |     set_param(gcb,'MaskEnables',{'on','off','on','on'}); % One setting for each parameter in this mask; needs to be updated if the parameters are changed
      | else
      |     % Enable the box
      |     set_param(gcb,'MaskEnables',{'on','on','on','on'}); % One setting for each parameter in this mask; needs to be updated if the parameters are changed
      | end
      mask Callback Limit:
      | % Author: Jakob Andersson (jande573)
      | 
      | % Define the default value to apply if the entered value is disallowed
      | default='1e-6';
      | 
      | % Evaluate the entered value
      | val=eval(get_param(gcb,'Limit'));
      | 
      | % Test the entered value and revert to the default if necessary
      | if isnumeric(val)==false
      |     % Set the default if the entered value did not evaluate to a numeric
      |     set_param(gcb,'Limit',default);
      |     errordlg('The limit must be numeric. The default value will be used instead.');
      | elseif val<0
      |     % Set the default if the entered value is negative
      |     set_param(gcb,'Limit',default);
      |     errordlg('The limit must be positive since it is compared to an absolute value. The default value will be used instead.');
      | end
      mask TypeOptions Operator:
      | Relational
      | Operator/Operator
      mask Callback Operator:
      | % Author: Jakob Andersson (jande573)
      | 
      | % Define the default value to apply if the entered value is disallowed
      | EnabledOperators={'==','~=','<','<=','>=','>'};
      | default='>';
      | 
      | % Evaluate the selected operator and determine if it is ok
      | ok=any(strcmp(get_param(gcb,'Operator'),EnabledOperators));
      | 
      | % Revert the operator if it is disabled
      | if ~ok
      |     % Set the default if the entered value did not evaluate to a numeric
      |     set_param(gcb,'Operator',default);
      |     errordlg('The selected operator is disabled. The default operator will be used instead.');
      | end
      mask Display:
      | % Display the mask for this block
      | % Author: Jakob Andersson (jande573)
      | 
      | % Transform the operator to TeX
      | switch Operator
      |     case '=='
      |         op='=';
      |     case '~='
      |         op='\neq';
      |     case '<='
      |         op='\leq';
      |     case '>='
      |         op='\geq';
      |     otherwise
      |         op=Operator;
      | end
      | 
      | % Display the correct mask depending on the number of inputs
      | if strcmp(UseInput,'on')
      |     % Display the block logic and the set limit
      |     disp(horzcat('|u| ',op,' a'),'texmode','on');
      | 
      |     % Display the port title
      |     port_label('input',1,'u');
      |     port_label('input',2,'a');
      | else
      |     % Display the block logic and the set limit
      |     disp(horzcat('|u| ',op,' ',num2str(Limit)),'texmode','on');
      | 
      |     % Display the port title
      |     port_label('input',1,'u');
      | end
  Square : Math  Operator=square  SignedPower=on  SaturateOnIntegerOverflow=on
  Switch : Switch  Criteria=u2 ~= 0
  NoSolution : Outport
  wires:
  Constant/1 -> Switch/3  "False"
  Switch/1 -> NoSolution/1  "NoSolution"
  SizeChecker/1(TestOk) -> Switch/2  "ARelIsDetectable"
  VRel/1 -> Square/1
  ARel/1 -> Compare To Zero/1(u), Compare To Zero1/1(u), SizeChecker/1(Signal), Gain/1
  EdgeDistance/1 -> Relop_1/1, Relop_2/1
  Square/1 -> LimitedSafeDivide/1(Numerator)
  Gain/1 -> LimitedSafeDivide/2(Denominator)
  LimitedSafeDivide/1(LimitedQuotient) -> Relop_1/2, Relop_2/2
  Compare To Zero/1(y) -> Logop_1/2  "PositiveARel"
  Relop_1/1 -> Logop_1/1
  Compare To Zero1/1(y) -> Logop_2/2  "NegativeARel"
  Logop_1/1 -> Logop_3/1  "NoSolutionPositiveARel"
  Logop_2/1 -> Logop_3/2  "NoSolutionNegativeARel"
  Logop_3/1 -> Switch/1  "NoSolutionCalculatedUsingARel"
  [BelowLimit] -> Logop_2/1
  Relop_2/1 -> [BelowLimit]
